simulink library "inp"  (Simulink 8.2) (continued)
== system inp/INP/InputSignals/DirectPassThrough == (continued)
  Cast230/1 -> Bus/69  "VINP_PassengerDoorOpenValid_flg"
  Bus Selector226/11 -> Cast229/1  "<VINP_PassengerDoorOpen_flg_InHAL>"
  Bus Selector226/12 -> Cast230/1  "<VINP_PassengerDoorOpenValid_flg_InHAL>"
  Cast231/1 -> Bus/70  "VINP_LRDoorOpen_flg"
  Cast232/1 -> Bus/71  "VINP_LRDoorOpenValid_flg"
  Bus Selector226/13 -> Cast231/1  "<VINP_LRDoorOpen_flg_InHAL>"
  Bus Selector226/14 -> Cast232/1  "<VINP_LRDoorOpenValid_flg_InHAL>"
  Cast4/1 -> Bus/72  "VINP_RRDoorOpen_flg"
  Cast5/1 -> Bus/73  "VINP_RRDoorOpenValid_flg"
  Bus Selector226/15 -> Cast4/1  "<VINP_RRDoorOpen_flg_InHAL>"
  Bus Selector226/16 -> Cast5/1  "<VINP_RRDoorOpenValid_flg_InHAL>"
  Cast13/1 -> Bus/74  "VINP_TailGateOpen_flg"
  Cast14/1 -> Bus/75  "VINP_TailGateOpenValid_flg"
  Bus Selector226/17 -> Cast13/1  "<VINP_TailGateOpen_flg_InHAL>"
  Bus Selector226/18 -> Cast14/1  "<VINP_TailGateOpenValid_flg_InHAL>"
  Cast15/1 -> Bus/76  "VINP_AnyDoorOpen_flg"
  Cast16/1 -> Bus/77  "VINP_AnyDoorOpenValid_flg"
  Bus Selector226/19 -> Cast15/1  "<VINP_AnyDoorOpen_flg_InHAL>"
  Bus Selector226/20 -> Cast16/1  "<VINP_AnyDoorOpenValid_flg_InHAL>"
  Cast17/1 -> Bus/78  "VINP_VehCrashed_flg"
  Cast18/1 -> Bus/79  "VINP_VehCrashedValid_flg"
  Bus Selector226/21 -> Cast17/1  "<VINP_VehCrashed_flg_InHAL>"
  Bus Selector226/22 -> Cast18/1  "<VINP_VehCrashedValid_flg_InHAL>"
  Cast19/1 -> Bus/96  "VINP_HSAActive_flg"
  Cast20/1 -> Bus/97  "VINP_HSAActiveValid_flg"
  Bus Selector209/17 -> Cast19/1  "<VINP_HSAActive_flg_InHAL>"
  Bus Selector209/18 -> Cast20/1  "<VINP_HSAActiveValid_flg_InHAL>"
  Cast21/1 -> Bus/98  "VINP_HSAFault_flg"
  Cast22/1 -> Bus/99  "VINP_HSAFaultValid_flg"
  Bus Selector209/19 -> Cast21/1  "<VINP_HSAFault_flg_InHAL>"
  Bus Selector209/20 -> Cast22/1  "<VINP_HSAFaultValid_flg_InHAL>"
  Cast23/1 -> Bus/113  "VINP_SurfaceGradeValid_flg"
  Cast24/1 -> Bus/112  "VINP_SurfaceGrade_deg"
  Bus Selector7/13 -> Cast24/1  "<VINP_SurfaceGrade_deg_InHAL>"
  Bus Selector7/14 -> Cast23/1  "<VINP_SurfaceGradeValid_flg_InHAL>"
  Cast25/1 -> Bus/115  "VINP_BrakBoosterPresValid_flg"
  Cast26/1 -> Bus/114  "VINP_BrakBoosterPres_kPa"
  Bus Selector7/15 -> Cast26/1  "<VINP_BrakBoosterPres_kPa_InHAL>"
  Bus Selector7/16 -> Cast25/1  "<VINP_BrakBoosterPresValid_flg_InHAL>"
  Cast27/1 -> Bus/116  "VINP_LowBrakBoosterPres_flg"
  Cast28/1 -> Bus/117  "VINP_LowBrakBoosterPresValid_flg"
  Bus Selector7/17 -> Cast27/1  "<VINP_LowBrakBoosterPres_flg_InHAL>"
  Bus Selector7/18 -> Cast28/1  "<VINP_LowBrakBoosterPresValid_flg_InHAL>"
  [ABS_signal_bus] -> Bus Selector3/1
  Cast34/1 -> Bus/119  "VINP_LFWheelSpeedValid_flg"
  Cast35/1 -> Bus/118  "VINP_LFWheelSpeed_kph"
  Bus Selector3/1 -> Cast35/1  "<VINP_LFWheelSpeed_kph_InHAL>"
  Bus Selector3/2 -> Cast34/1  "<VINP_LFWheelSpeedValid_flg_InHAL>"
  Cast36/1 -> Bus/121  "VINP_LRWheelSpeedValid_flg"
  Cast37/1 -> Bus/120  "VINP_LRWheelSpeed_kph"
  Bus Selector3/3 -> Cast37/1  "<VINP_LRWheelSpeed_kph_InHAL>"
  Bus Selector3/4 -> Cast36/1  "<VINP_LRWheelSpeedValid_flg_InHAL>"
  Cast38/1 -> Bus/123  "VINP_RFWheelSpeedValid_flg"
  Cast39/1 -> Bus/122  "VINP_RFWheelSpeed_kph"
  Bus Selector3/5 -> Cast39/1  "<VINP_RFWheelSpeed_kph_InHAL>"
  Bus Selector3/6 -> Cast38/1  "<VINP_RFWheelSpeedValid_flg_InHAL>"
  Cast40/1 -> Bus/125  "VINP_RRWheelSpeedValid_flg"
  Cast41/1 -> Bus/124  "VINP_RRWheelSpeed_kph"
  Bus Selector3/7 -> Cast41/1  "<VINP_RRWheelSpeed_kph_InHAL>"
  Bus Selector3/8 -> Cast40/1  "<VINP_RRWheelSpeedValid_flg_InHAL>"
  Cast42/1 -> Bus/131  "VINP_RelSteerAngleValid_flg"
  Cast44/1 -> Bus/130  "VINP_RelSteerAngle_deg"
  Bus Selector232/1 -> Cast44/1  "<VINP_RelSteerAngle_deg_InHAL>"
  Bus Selector232/2 -> Cast42/1  "<VINP_RelSteerAngleValid_flg_InHAL>"
  Cast151/1 -> Bus/136  "VINP_EPSFailed_flg"
  Cast152/1 -> Bus/137  "VINP_EPSFailedValid_flg"
  Bus Selector232/7 -> Cast151/1  "<VINP_EPSFailed_flg_InHAL>"
  Bus Selector232/8 -> Cast152/1  "<VINP_EPSFailedValid_flg_InHAL>"
  Cast153/1 -> Bus/139  "VINP_AckCurStrAngReqValid_flg"
  Cast154/1 -> Bus/138  "VINP_AckCurStrAngReq_deg"
  Bus Selector232/9 -> Cast154/1  "<VINP_AckCurStrAngReq_deg_InHAL>"
  Bus Selector232/10 -> Cast153/1  "<VINP_AckCurStrAngReqValid_flg_InHAL>"
  Cast155/1 -> Bus/140  "VINP_AckCurStrAngReqApplable_flg"
  Cast156/1 -> Bus/141  "VINP_AckCurStrAngReqApplableValid_flg"
  Bus Selector232/11 -> Cast155/1  "<VINP_AckCurStrAngReqApplable_flg_InHAL>"
  Bus Selector232/12 -> Cast156/1  "<VINP_AckCurStrAngReqApplableValid_flg_InHAL>"
  Cast157/1 -> Bus/143  "VINP_AckCurStrTqReqValid_flg"
  Cast158/1 -> Bus/142  "VINP_AckCurStrTqReq_Nm"
  Bus Selector232/13 -> Cast158/1  "<VINP_AckCurStrTqReq_Nm_InHAL>"
  Bus Selector232/14 -> Cast157/1  "<VINP_AckCurStrTqReqValid_flg_InHAL>"
  Cast159/1 -> Bus/144  "VINP_AckCurStrTqReqApplable_flg"
  Cast160/1 -> Bus/145  "VINP_AckCurStrTqReqApplableValid_flg"
  Bus Selector232/15 -> Cast159/1  "<VINP_AckCurStrTqReqApplable_flg_InHAL>"
  Bus Selector232/16 -> Cast160/1  "<VINP_AckCurStrTqReqApplableValid_flg_InHAL>"
  Cast161/1 -> Bus/147  "VINP_EPSBattVoltValid_flg"
  Cast162/1 -> Bus/146  "VINP_EPSBattVolt_V"
  Bus Selector232/17 -> Cast162/1  "<VINP_EPSBattVolt_V_InHAL>"
  Bus Selector232/18 -> Cast161/1  "<VINP_EPSBattVoltValid_flg_InHAL>"
  [METER_signal_bus] -> Bus Selector6/1
  Cast167/1 -> Bus/157  "VINP_OdoMeterValid_flg"
  Cast168/1 -> Bus/156  "VINP_OdoMeter_km"
  Bus Selector6/1 -> Cast168/1  "<VINP_OdoMeter_km_InHAL>"
  Bus Selector6/2 -> Cast167/1  "<VINP_OdoMeterValid_flg_InHAL>"
  Cast169/1 -> Bus/158  "VINP_LowBrakeFluid_flg"
  Cast170/1 -> Bus/159  "VINP_LowBrakeFluidValid_flg"
  Bus Selector6/3 -> Cast169/1  "<VINP_LowBrakeFluid_flg_InHAL>"
  Bus Selector6/4 -> Cast170/1  "<VINP_LowBrakeFluidValid_flg_InHAL>"
  Cast171/1 -> Bus/160  "VINP_ParkBrakeOn_flg"
  Cast172/1 -> Bus/161  "VINP_ParkBrakeOnValid_flg"
  Bus Selector6/5 -> Cast171/1  "<VINP_ParkBrakeOn_flg_InHAL>"
  Bus Selector6/6 -> Cast172/1  "<VINP_ParkBrakeOnValid_flg_InHAL>"
  Cast173/1 -> Bus/162  "VINP_SeatBeltNOTFasten_flg"
  Cast174/1 -> Bus/163  "VINP_SeatBeltNOTFastenValid_flg"
  Bus Selector6/7 -> Cast173/1  "<VINP_SeatBeltNOTFasten_flg_InHAL>"
  Bus Selector6/8 -> Cast174/1  "<VINP_SeatBeltNOTFastenValid_flg_InHAL>"
  Cast175/1 -> Bus/165  "VINP_MeterVehSpdValid_flg"
  Cast176/1 -> Bus/164  "VINP_MeterVehSpd_kph"
  Bus Selector6/9 -> Cast176/1  "<VINP_MeterVehSpd_kph_InHAL>"
  Bus Selector6/10 -> Cast175/1  "<VINP_MeterVehSpdValid_flg_InHAL>"
  [AC_signal_bus] -> Bus Selector8/1
  Cast177/1 -> Bus/167  "VINP_OutsideTempValid_flg"
  Cast178/1 -> Bus/166  "VINP_OutsideTemp_degC"
  Bus Selector8/1 -> Cast178/1  "<VINP_OutsideTemp_degC_InHAL>"
  Bus Selector8/2 -> Cast177/1  "<VINP_OutsideTempValid_flg_InHAL>"
  [EPB_signal_bus] -> Bus Selector9/1
  Bus Selector9/1 -> Cast179/1  "<VINP_ParkBrakeBindSta_enum_InHAL>"
  Bus Selector9/2 -> Cast180/1  "<VINP_ParkBrakeBindStaValid_flg_InHAL>"
  Cast179/1 -> Bus/168  "VINP_ParkBrakeBindSta_enum"
  Cast180/1 -> Bus/169  "VINP_ParkBrakeBindStaValid_flg"
  [VCU_signal_bus] -> Bus Selector10/1
  Cast233/1 -> Bus/174  "VINP_AckCurBrakDecReq_mps2"
  Cast234/1 -> Bus/175  "VINP_AckCurBrakDecReqValid_flg"
  Bus Selector10/1 -> Cast233/1  "<VINP_AckCurBrakDecReq_mps2_InHAL>"
  Bus Selector10/2 -> Cast234/1  "<VINP_AckCurBrakDecReqValid_flg_InHAL>"
  Cast235/1 -> Bus/176  "VINP_AckCurBrakDecReqApplable_flg"
  Cast236/1 -> Bus/177  "VINP_AckCurBrakDecReqApplableValid_flg"
  Bus Selector10/3 -> Cast235/1  "<VINP_AckCurBrakDecReqApplable_flg_InHAL>"
  Bus Selector10/4 -> Cast236/1  "<VINP_AckCurBrakDecReqApplableValid_flg_InHAL>"
  Cast237/1 -> Bus/178  "VINP_AckCurPTTqReq_Nm"
  Cast238/1 -> Bus/179  "VINP_AckCurPTTqReqValid_flg"
  Cast239/1 -> Bus/180  "VINP_AckCurPTTqReqApplable_flg"
  Cast240/1 -> Bus/181  "VINP_AckCurPTTqReqApplableValid_flg"
  Bus Selector10/5 -> Cast237/1  "<VINP_AckCurPTTqReq_Nm_InHAL>"
  Bus Selector10/6 -> Cast238/1  "<VINP_AckCurPTTqReqValid_flg_InHAL>"
  Bus Selector10/7 -> Cast239/1  "<VINP_AckCurPTTqReqApplable_flg_InHAL>"
  Bus Selector10/8 -> Cast240/1  "<VINP_AckCurPTTqReqApplableValid_flg_InHAL>"
  Bus Selector10/9 -> Cast241/1  "<VINP_AckCurVehDec_mps2_InHAL>"
  Cast241/1 -> Bus/182  "VINP_AckCurVehDec_mps2"
  Cast242/1 -> Bus/183  "VINP_AckCurVehDecValid_flg"
  Bus Selector10/10 -> Cast242/1  "<VINP_AckCurVehDecValid_flg_InHAL>"
  Bus Selector10/11 -> Cast245/1  "<VINP_DeceContManag_flg_InHAL>"
  Cast245/1 -> Bus/184  "VINP_DeceContManag_flg"
  Cast246/1 -> Bus/185  "VINP_DeceContManagValid_flg"
  Bus Selector10/12 -> Cast246/1  "<VINP_DeceContManagValid_flg_InHAL>"
  Bus Selector10/13 -> Cast247/1  "<VINP_DriverBrakPedalOn_flg_InHAL>"
  Cast247/1 -> Bus/186  "VINP_DriverBrakPedalOn_flg"
  Cast248/1 -> Bus/187  "VINP_DriverBrakPedalOnValid_flg"
  Bus Selector10/14 -> Cast248/1  "<VINP_DriverBrakPedalOnValid_flg_InHAL>"
  Bus Selector10/15 -> Cast249/1  "<VINP_ECMExtnTqAvailable_flg_InHAL>"
  Cast249/1 -> Bus/188  "VINP_ECMExtnTqAvailable_flg"
  Cast250/1 -> Bus/189  "VINP_ECMExtnTqAvailableValid_flg"
  Bus Selector10/16 -> Cast250/1  "<VINP_ECMExtnTqAvailableValid_flg_InHAL>"
  Bus Selector10/17 -> Cast251/1  "<VINP_ECMTqSource_enum_InHAL>"
  Cast251/1 -> Bus/190  "VINP_ECMTqSource_enum"
  Cast252/1 -> Bus/191  "VINP_ECMTqSourceValid_flg"
  Bus Selector10/18 -> Cast252/1  "<VINP_ECMTqSourceValid_flg_InHAL>"
  Bus Selector10/19 -> Cast253/1  "<VINP_ESCBrakeStatus_enum_InHAL>"
  Cast253/1 -> Bus/192  "VINP_ESCBrakeStatus_enum"
  Cast254/1 -> Bus/193  "VINP_ESCBrakeStatusValid_flg"
  Bus Selector10/20 -> Cast254/1  "<VINP_ESCBrakeStatusValid_flg_InHAL>"
  Bus Selector10/21 -> Cast255/1  "<VINP_ESCExtnBrkAvailable_flg_InHAL>"
  Cast255/1 -> Bus/194  "VINP_ESCExtnBrkAvailable_flg"
  Cast256/1 -> Bus/195  "VINP_ESCExtnBrkAvailableValid_flg"
  Bus Selector10/22 -> Cast256/1  "<VINP_ESCExtnBrkAvailableValid_flg_InHAL>"
  Bus Selector10/23 -> Cast257/1  "<VINP_ESCBattVolt_V_InHAL>"
  Cast257/1 -> Bus/196  "VINP_ESCBattVolt_V"
  Cast258/1 -> Bus/197  "VINP_ESCBattVoltValid_flg"
  Bus Selector10/24 -> Cast258/1  "<VINP_ESCBattVoltValid_flg_InHAL>"
  Bus Selector10/25 -> Cast259/1  "<VINP_HWAccelPedalPosn_pct_InHAL>"
  Cast259/1 -> Bus/198  "VINP_HWAccelPedalPosn_pct"
  Cast260/1 -> Bus/199  "VINP_HWAccelPedalPosnValid_flg"
  Bus Selector10/26 -> Cast260/1  "<VINP_HWAccelPedalPosnValid_flg_InHAL>"
  Cast263/1 -> Bus/208  "VINP_HCUMILReq_flg"
  Cast264/1 -> Bus/209  "VINP_HCUMILReqValid_flg"
  [VCU_signal_bus] -> Bus Selector11/1
  Cast271/1 -> Bus/218  "VINP_ACCTimeGapIncVCU_flg"
  Cast272/1 -> Bus/219  "VINP_ACCTimeGapIncVCUValid_flg"
  Bus Selector11/1 -> Cast271/1  "<VINP_ACCTimeGapIncVCU_flg_InHAL>"
  Bus Selector11/2 -> Cast272/1  "<VINP_ACCTimeGapIncVCUValid_flg_InHAL>"
  Cast273/1 -> Bus/220  "VINP_ACCTimeGapDecVCU_flg"
  Cast274/1 -> Bus/221  "VINP_ACCTimeGapDecVCUValid_flg"
  Bus Selector11/3 -> Cast273/1  "<VINP_ACCTimeGapDecVCU_flg_InHAL>"
  Bus Selector11/4 -> Cast274/1  "<VINP_ACCTimeGapDecVCUValid_flg_InHAL>"
  Cast275/1 -> Bus/222  "VINP_LFCOnVCU_flg"
  Cast276/1 -> Bus/223  "VINP_LFCOnVCUValid_flg"
  [HMI_signal_bus] -> Bus Selector12/1
  Cast277/1 -> Bus/226  "VINP_AEBEnableH_flg"
  Cast278/1 -> Bus/227  "VINP_AEBEnableHValid_flg"
  Bus Selector12/1 -> Cast277/1  "<VINP_AEBEnableH_flg_InHAL>"
  Bus Selector12/2 -> Cast278/1  "<VINP_AEBEnableHValid_flg_InHAL>"
  Cast279/1 -> Bus/228  "VINP_FCWEnableH_flg"
  Cast280/1 -> Bus/229  "VINP_FCWEnableHValid_flg"
  Bus Selector12/3 -> Cast279/1  "<VINP_FCWEnableH_flg_InHAL>"
  Bus Selector12/4 -> Cast280/1  "<VINP_FCWEnableHValid_flg_InHAL>"
  Cast281/1 -> Bus/230  "VINP_CSCEnableH_flg"
  Bus Selector12/5 -> Cast281/1  "<VINP_CSCEnableH_flg_InHAL>"
  Cast282/1 -> Bus/231  "VINP_CSCEnableHValid_flg"
  Bus Selector12/6 -> Cast282/1  "<VINP_CSCEnableHValid_flg_InHAL>"
  Cast283/1 -> Bus/232  "VINP_LKAEnableH_flg"
  Cast284/1 -> Bus/233  "VINP_LKAEnableHValid_flg"
  Bus Selector12/7 -> Cast283/1  "<VINP_LKAEnableH_flg_InHAL>"
  Bus Selector12/8 -> Cast284/1  "<VINP_LKAEnableHValid_flg_InHAL>"
  Cast285/1 -> Bus/234  "VINP_MAPAEnableH_flg"
  Cast286/1 -> Bus/235  "VINP_MAPAEnableHValid_flg"
  Bus Selector12/9 -> Cast285/1  "<VINP_MAPAEnableH_flg_InHAL>"
  Bus Selector12/10 -> Cast286/1  "<VINP_MAPAEnableHValid_flg_InHAL>"
  Cast287/1 -> Bus/236  "VINP_LCSEnableH_flg"
  Cast288/1 -> Bus/237  "VINP_LCSEnableHValid_flg"
  Bus Selector12/11 -> Cast287/1  "<VINP_LCSEnableH_flg_InHAL>"
  Bus Selector12/12 -> Cast288/1  "<VINP_LCSEnableHValid_flg_InHAL>"
  [HMI_signal_bus] -> Bus Selector13/1
  [INP_calc_bus] -> Bus Selector14/1
  Cast289/1 -> Bus/242  "VINP_LFCPilotOnH_flg"
  Cast290/1 -> Bus/243  "VINP_LFCPilotOnHValid_flg"
  Bus Selector14/1 -> Cast289/1  "<VINP_LFCPilotOnH_flg_InProc>"
  Bus Selector13/1 -> Cast290/1  "<VINP_LFCPilotOnHValid_flg_InHAL>"
  [HMI_signal_bus] -> Bus Selector15/1
  [INP_calc_bus] -> Bus Selector16/1
  Cast291/1 -> Bus/244  "VINP_LFCPilotCancelH_flg"
  Cast292/1 -> Bus/245  "VINP_LFCPilotCancelHValid_flg"
  Bus Selector16/1 -> Cast291/1  "<VINP_LFCPilotCancelH_flg_InProc>"
  Bus Selector15/1 -> Cast292/1  "<VINP_LFCPilotCancelHValid_flg_InHAL>"
  [HMI_signal_bus] -> Bus Selector17/1
  [INP_calc_bus] -> Bus Selector18/1
  Cast293/1 -> Bus/246  "VINP_ACCOnH_flg"
  Cast294/1 -> Bus/247  "VINP_ACCOnHValid_flg"
  Bus Selector18/1 -> Cast293/1  "<VINP_ACCOnH_flg_InProc>"
  Bus Selector17/1 -> Cast294/1  "<VINP_ACCOnHValid_flg_InHAL>"
  [HMI_signal_bus] -> Bus Selector19/1
  [INP_calc_bus] -> Bus Selector20/1
  Cast295/1 -> Bus/248  "VINP_ACCCancelH_flg"
  Cast296/1 -> Bus/249  "VINP_ACCCancelHValid_flg"
  Bus Selector20/1 -> Cast295/1  "<VINP_ACCCancelH_flg_InProc>"
  Bus Selector19/1 -> Cast296/1  "<VINP_ACCCancelHValid_flg_InHAL>"
  [HMI_signal_bus] -> Bus Selector21/1
  [INP_calc_bus] -> Bus Selector22/1
  Cast297/1 -> Bus/250  "VINP_ACCResSpdIncH_flg"
  Cast298/1 -> Bus/251  "VINP_ACCResSpdIncHValid_flg"
  Bus Selector22/1 -> Cast297/1  "<VINP_ACCResSpdIncH_flg_InProc>"
  Bus Selector21/1 -> Cast298/1  "<VINP_ACCResSpdIncHValid_flg_InHAL>"
  [HMI_signal_bus] -> Bus Selector23/1
  [INP_calc_bus] -> Bus Selector24/1
  Cast299/1 -> Bus/252  "VINP_ACCSetSpdDecH_flg"
  Cast300/1 -> Bus/253  "VINP_ACCSetSpdDecHValid_flg"
  Bus Selector24/1 -> Cast299/1  "<VINP_ACCSetSpdDecH_flg_InProc>"
  Bus Selector23/1 -> Cast300/1  "<VINP_ACCSetSpdDecHValid_flg_InHAL>"
  [HMI_signal_bus] -> Bus Selector25/1
  [INP_calc_bus] -> Bus Selector26/1
  Cast301/1 -> Bus/254  "VINP_ACCSpdIncH_flg"
  Cast302/1 -> Bus/255  "VINP_ACCSpdIncHValid_flg"
  Bus Selector26/1 -> Cast301/1  "<VINP_ACCSpdIncH_flg_InProc>"
  Bus Selector25/1 -> Cast302/1  "<VINP_ACCSpdIncHValid_flg_InHAL>"
  [HMI_signal_bus] -> Bus Selector27/1
  [INP_calc_bus] -> Bus Selector28/1
  Cast303/1 -> Bus/256  "VINP_ACCSpdDecH_flg"
  Cast304/1 -> Bus/257  "VINP_ACCSpdDecHValid_flg"
  Bus Selector28/1 -> Cast303/1  "<VINP_ACCSpdDecH_flg_InProc>"
  Bus Selector27/1 -> Cast304/1  "<VINP_ACCSpdDecHValid_flg_InHAL>"
  [HMI_signal_bus] -> Bus Selector29/1
  [INP_calc_bus] -> Bus Selector30/1
  Cast305/1 -> Bus/258  "VINP_ACCTimeGapIncH_flg"
  Cast306/1 -> Bus/259  "VINP_ACCTimeGapIncHValid_flg"
  Bus Selector30/1 -> Cast305/1  "<VINP_ACCTimeGapIncH_flg_InProc>"
  Bus Selector29/1 -> Cast306/1  "<VINP_ACCTimeGapIncHValid_flg_InHAL>"
  [HMI_signal_bus] -> Bus Selector31/1
  [INP_calc_bus] -> Bus Selector32/1
  Cast307/1 -> Bus/260  "VINP_ACCTimeGapDecH_flg"
  Cast308/1 -> Bus/261  "VINP_ACCTimeGapDecHValid_flg"
  Bus Selector32/1 -> Cast307/1  "<VINP_ACCTimeGapDecH_flg_InProc>"
  Bus Selector31/1 -> Cast308/1  "<VINP_ACCTimeGapDecHValid_flg_InHAL>"
  [HMI_signal_bus] -> Bus Selector33/1
  [INP_calc_bus] -> Bus Selector34/1
  Cast309/1 -> Bus/262  "VINP_IGSAOnH_flg"
  Cast310/1 -> Bus/263  "VINP_IGSAOnHValid_flg"
  Bus Selector34/1 -> Cast309/1  "<VINP_IGSAOnH_flg_InProc>"
  Bus Selector33/1 -> Cast310/1  "<VINP_IGSAOnHValid_flg_InHAL>"
  [HMI_signal_bus] -> Bus Selector35/1
  [INP_calc_bus] -> Bus Selector36/1
  Cast311/1 -> Bus/264  "VINP_IGSAResSpdIncH_flg"
  Cast312/1 -> Bus/265  "VINP_IGSAResSpdIncHValid_flg"
  Bus Selector36/1 -> Cast311/1  "<VINP_IGSAResSpdIncH_flg_InProc>"
  Bus Selector35/1 -> Cast312/1  "<VINP_IGSAResSpdIncHValid_flg_InHAL>"
  [HMI_signal_bus] -> Bus Selector37/1
  [INP_calc_bus] -> Bus Selector38/1
  Cast313/1 -> Bus/266  "VINP_IGSASetSpdDecH_flg"
  Cast314/1 -> Bus/267  "VINP_IGSASetSpdDecHValid_flg"
  Bus Selector38/1 -> Cast313/1  "<VINP_IGSASetSpdDecH_flg_InProc>"
  Bus Selector37/1 -> Cast314/1  "<VINP_IGSASetSpdDecHValid_flg_InHAL>"
  [HMI_signal_bus] -> Bus Selector39/1
  [INP_calc_bus] -> Bus Selector40/1
  Cast315/1 -> Bus/268  "VINP_IGSASwitchModeH_flg"
  Cast316/1 -> Bus/269  "VINP_IGSASwitchModeHValid_flg"
  Bus Selector40/1 -> Cast315/1  "<VINP_IGSASwitchModeH_flg_InProc>"
  Bus Selector39/1 -> Cast316/1  "<VINP_IGSASwitchModeHValid_flg_InHAL>"
  Cast64/1 -> Bus/442  "VINP_ABSCANValid_flg"
  Cast65/1 -> Bus/454  "VINP_ABSCANAlive_flg"
  Cast68/1 -> Bus/443  "VINP_BCMCANValid_flg"
  Cast69/1 -> Bus/455  "VINP_BCMCANAlive_flg"
  Cast321/1 -> Bus/444  "VINP_ECMCANValid_flg"
  Cast322/1 -> Bus/456  "VINP_ECMCANAlive_flg"
  Cast323/1 -> Bus/445  "VINP_TCMCANValid_flg"
  Cast324/1 -> Bus/457  "VINP_TCMCANAlive_flg"
  Cast325/1 -> Bus/446  "VINP_EPSCANValid_flg"
  Cast326/1 -> Bus/458  "VINP_EPSCANAlive_flg"
  [INP_diag_bus] -> Bus Selector241/1
  Bus Selector241/1 -> Cast64/1  "<VINP_ABSCANValid_flg_InProc>"
  [INP_diag_bus] -> Bus Selector242/1
  Bus Selector242/1 -> Cast65/1  "<VINP_ABSCANAlive_flg_InProc>"
  Bus Selector241/2 -> Cast68/1  "<VINP_BCMCANValid_flg_InProc>"
  Bus Selector242/2 -> Cast69/1  "<VINP_BCMCANAlive_flg_InProc>"
  Bus Selector241/3 -> Cast321/1  "<VINP_ECMCANValid_flg_InProc>"
  Bus Selector242/3 -> Cast322/1  "<VINP_ECMCANAlive_flg_InProc>"
  Bus Selector241/4 -> Cast323/1  "<VINP_TCMCANValid_flg_InProc>"
  Bus Selector242/4 -> Cast324/1  "<VINP_TCMCANAlive_flg_InProc>"
  Bus Selector241/5 -> Cast325/1  "<VINP_EPSCANValid_flg_InProc>"
  Bus Selector242/5 -> Cast326/1  "<VINP_EPSCANAlive_flg_InProc>"
  Cast56/1 -> Bus/447  "VINP_METERCANValid_flg"
  Bus Selector241/6 -> Cast56/1  "<VINP_METERCANValid_flg_InProc>"
  Cast57/1 -> Bus/448  "VINP_ACCANValid_flg"
  Bus Selector241/7 -> Cast57/1  "<VINP_ACCANValid_flg_InProc>"
  Cast58/1 -> Bus/449  "VINP_EPBCANValid_flg"
  Bus Selector241/8 -> Cast58/1  "<VINP_EPBCANValid_flg_InProc>"
  Cast59/1 -> Bus/450  "VINP_VCUCANValid_flg"
  Bus Selector241/9 -> Cast59/1  "<VINP_VCUCANValid_flg_InProc>"
  Cast60/1 -> Bus/459  "VINP_METERCANAlive_flg"
  Bus Selector242/6 -> Cast60/1  "<VINP_METERCANAlive_flg_InProc>"
  Cast61/1 -> Bus/460  "VINP_ACCANAlive_flg"
  Bus Selector242/7 -> Cast61/1  "<VINP_ACCANAlive_flg_InProc>"
  Cast62/1 -> Bus/461  "VINP_EPBCANAlive_flg"
  Bus Selector242/8 -> Cast62/1  "<VINP_EPBCANAlive_flg_InProc>"
  Cast63/1 -> Bus/462  "VINP_VCUCANAlive_flg"
  Bus Selector242/9 -> Cast63/1  "<VINP_VCUCANAlive_flg_InProc>"
  Cast327/1 -> Bus/451  "VINP_HMICANValid_flg"
  Bus Selector241/10 -> Cast327/1  "<VINP_HMICANValid_flg_InProc>"
  Cast328/1 -> Bus/453  "VINP_EPMCANValid_flg"
  Bus Selector241/12 -> Cast328/1  "<VINP_EPMCANValid_flg_InProc>"
  Cast329/1 -> Bus/463  "VINP_HMICANAlive_flg"
  Bus Selector242/10 -> Cast329/1  "<VINP_HMICANAlive_flg_InProc>"
  Cast330/1 -> Bus/465  "VINP_EPMCANAlive_flg"
  Bus Selector242/12 -> Cast330/1  "<VINP_EPMCANAlive_flg_InProc>"
  Cast331/1 -> Bus/3  "VINP_BatteryVolt_V"
  Cast332/1 -> Bus/4  "VINP_BatteryVoltValid_flg"
  Bus Selector197/3 -> Cast331/1  "<VINP_BatteryVolt_V_InHAL>"
  Bus Selector197/4 -> Cast332/1  "<VINP_BatteryVoltValid_flg_InHAL>"
  Cast334/1 -> Bus/129  "VINP_SteerSpeedValid_flg"
  Cast333/1 -> Bus/128  "VINP_SteerSpeed_degps"
  Bus Selector42/1 -> Cast333/1  "<VINP_SteerSpeed_degps_InProc>"
  Bus Selector42/2 -> Cast334/1  "<VINP_SteerSpeedValid_flg_InProc>"
  Cast335/1 -> Bus/148  "VINP_EPASMILReq_flg"
  Bus Selector232/19 -> Cast335/1  "<VINP_EPASMILReq_flg_InHAL>"
  Cast336/1 -> Bus/149  "VINP_EPASMILReqValid_flg"
  Bus Selector232/20 -> Cast336/1  "<VINP_EPASMILReqValid_flg_InHAL>"
  Cast337/1 -> Bus/200  "VINP_CurDriverTqReq_Nm"
  Cast338/1 -> Bus/201  "VINP_CurDriverTqReqValid_flg"
  Bus Selector10/27 -> Cast337/1  "<VINP_CurDriverTqReq_Nm_InHAL>"
  Bus Selector10/28 -> Cast338/1  "<VINP_CurDriverTqReqValid_flg_InHAL>"
  Cast339/1 -> Bus/238  "VINP_FCWWarningModeH_enum"
  Cast340/1 -> Bus/239  "VINP_FCWWarningModeHValid_flg"
  Bus Selector12/13 -> Cast339/1  "<VINP_FCWWarningModeH_enum_InHAL>"
  Bus Selector12/14 -> Cast340/1  "<VINP_FCWWarningModeHValid_flg_InHAL>"
  [EPS_signal_bus] -> Bus Selector41/1
  [INP_calc_bus] -> Bus Selector42/1
  Cast341/1 -> Bus/224  "VINP_MSAOnVCU_flg"
  Cast342/1 -> Bus/225  "VINP_MSAOnVCUValid_flg"
  Cast343/1 -> Bus/240  "VINP_DefMaxACCSetSpeed_kph"
  Cast344/1 -> Bus/241  "VINP_DefMaxACCSetSpeedValid_flg"
  Bus Selector12/15 -> Cast343/1  "<VINP_DefMaxACCSetSpeed_kph_InHAL>"
  Bus Selector12/16 -> Cast344/1  "<VINP_DefMaxACCSetSpeedValid_flg_InHAL>"
  [CSW_signal_bus] -> Terminator13/1
  CSW_signal_bus/1 -> [CSW_signal_bus]
  Bus Selector204/25 -> Cast1/1  "<VINP_OBDWarnCycAchiv_flg_InHAL>"
  Cast1/1 -> Bus/36  "VINP_OBDWarnCycAchiv_flg"
  Cast66/1 -> Bus/37  "VINP_OBDWarnCycAchivValid_flg"
  Bus Selector204/26 -> Cast66/1  "<VINP_OBDWarnCycAchivValid_flg_InHAL>"
  Cast67/1 -> Bus/38  "VINP_OBDDriveCycAchiv_flg"
  Cast78/1 -> Bus/39  "VINP_OBDDriveCycAchivValid_flg"
  Bus Selector204/27 -> Cast67/1  "<VINP_OBDDriveCycAchiv_flg_InHAL>"
  Bus Selector204/28 -> Cast78/1  "<VINP_OBDDriveCycAchivValid_flg_InHAL>"
  Bus Selector230/9 -> Cast141/1  "<VINP_TqConvClutchSt_enum_InHAL>"
  Cast141/1 -> Bus/48  "VINP_TqConvClutchSt_enum"
  Cast142/1 -> Bus/49  "VINP_TqConvClutchStValid_flg"
  Bus Selector230/10 -> Cast142/1  "<VINP_TqConvClutchStValid_flg_InHAL>"
  Bus Selector230/11 -> Cast182/1  "<VINP_TCMMILReq_flg_InHAL>"
  Cast182/1 -> Bus/50  "VINP_TCMMILReq_flg"
  Cast184/1 -> Bus/51  "VINP_TCMMILReqValid_flg"
  Bus Selector230/12 -> Cast184/1  "<VINP_TCMMILReqValid_flg_InHAL>"
  Bus Selector230/13 -> Cast185/1  "<VINP_TCMWinterMode_flg_InHAL>"
  Cast185/1 -> Bus/52  "VINP_TCMWinterMode_flg"
  Cast200/1 -> Bus/53  "VINP_TCMWinterModeValid_flg"
  Bus Selector230/14 -> Cast200/1  "<VINP_TCMWinterModeValid_flg_InHAL>"
  Cast201/1 -> Bus/54  "VINP_TCMPowerMode_flg"
  Cast224/1 -> Bus/55  "VINP_TCMPowerModeValid_flg"
  Bus Selector230/15 -> Cast201/1  "<VINP_TCMPowerMode_flg_InHAL>"
  Bus Selector230/16 -> Cast224/1  "<VINP_TCMPowerModeValid_flg_InHAL>"
  Cast30/1 -> Bus/150  "VINP_EPSLKAStatus_enum"
  Bus Selector232/21 -> Cast30/1  "<VINP_EPSLKAStatus_enum_InHAL>"
  Cast32/1 -> Bus/151  "VINP_EPSLKAStatusValid_flg"
  Bus Selector232/22 -> Cast32/1  "<VINP_EPSLKAStatusValid_flg_InHAL>"
  Cast163/1 -> Bus/152  "VINP_EPSLFCStatus_enum"
  Cast164/1 -> Bus/153  "VINP_EPSLFCStatusValid_flg"
  Bus Selector232/23 -> Cast163/1  "<VINP_EPSLFCStatus_enum_InHAL>"
  Bus Selector232/24 -> Cast164/1  "<VINP_EPSLFCStatusValid_flg_InHAL>"
  Cast165/1 -> Bus/154  "VINP_EPSADASAbReason_enum"
  Cast166/1 -> Bus/155  "VINP_EPSADASAbReasonValid_flg"
  Bus Selector232/25 -> Cast165/1  "<VINP_EPSADASAbReason_enum_InHAL>"
  Bus Selector232/26 -> Cast166/1  "<VINP_EPSADASAbReasonValid_flg_InHAL>"
  [CSW_signal_bus] -> Bus Selector43/1
  Cast225/1 -> Bus/170  "VINP_RearFogSwOn_flg"
  Cast345/1 -> Bus/171  "VINP_RearFogSwOnValid_flg"
  Cast346/1 -> Bus/172  "VINP_HighBeamSwOn_flg"
  Cast347/1 -> Bus/173  "VINP_HighBeamSwOnValid_flg"
  Bus Selector43/1 -> Cast346/1  "<VINP_HighBeamSwOn_flg_InHAL>"
  Bus Selector43/2 -> Cast347/1  "<VINP_HighBeamSwOnValid_flg_InHAL>"
  Bus Selector10/35 -> Cast263/1  "<VINP_HCUMILReq_flg_InHAL>"
  Bus Selector10/36 -> Cast264/1  "<VINP_HCUMILReqValid_flg_InHAL>"
  Bus Selector10/29 -> Cast243/1  "<VINP_TCMGearPosnReqAllow_enum_InHAL>"
  Cast243/1 -> Bus/202  "VINP_TCMGearPosnReqAllow_enum"
  Cast244/1 -> Bus/203  "VINP_TCMGearPosnReqAllowValid_flg"
  Bus Selector10/30 -> Cast244/1  "<VINP_TCMGearPosnReqAllowValid_flg_InHAL>"
  Cast261/1 -> Bus/204  "VINP_MSAStatus_enum"
  Cast262/1 -> Bus/205  "VINP_MSAStatusValid_flg"
  Bus Selector10/31 -> Cast261/1  "<VINP_MSAStatus_enum_InHAL>"
  Bus Selector10/32 -> Cast262/1  "<VINP_MSAStatusValid_flg_InHAL>"
  Cast348/1 -> Bus/206  "VINP_HybridLimpHome_flg"
  Cast349/1 -> Bus/207  "VINP_HybridLimpHomeValid_flg"
  Bus Selector10/33 -> Cast348/1  "<VINP_HybridLimpHome_flg_InHAL>"
  Bus Selector10/34 -> Cast349/1  "<VINP_HybridLimpHomeValid_flg_InHAL>"
  [VCU_signal_bus] -> Bus Selector44/1
  Cast350/1 -> Bus/210  "VINP_ACCOnVCU_flg"
  Cast351/1 -> Bus/211  "VINP_ACCOnVCUValid_flg"
  Bus Selector45/1 -> Cast350/1  "<VINP_ACCOnVCU_flg_InProc>"
  Bus Selector45/2 -> Cast351/1  "<VINP_ACCOnVCUValid_flg_InProc>"
  Cast352/1 -> Bus/212  "VINP_ACCResSpdIncVCU_flg"
  Cast353/1 -> Bus/213  "VINP_ACCResSpdIncVCUValid_flg"
  Bus Selector44/1 -> Cast352/1  "<VINP_ACCResSpdIncVCU_flg_InHAL>"
  Bus Selector44/2 -> Cast353/1  "<VINP_ACCResSpdIncVCUValid_flg_InHAL>"
  Cast354/1 -> Bus/214  "VINP_ACCSetSpdDecVCU_flg"
  Cast355/1 -> Bus/215  "VINP_ACCSetSpdDecVCUValid_flg"
  Bus Selector44/3 -> Cast354/1  "<VINP_ACCSetSpdDecVCU_flg_InHAL>"
  Bus Selector44/4 -> Cast355/1  "<VINP_ACCSetSpdDecVCUValid_flg_InHAL>"
  Cast356/1 -> Bus/216  "VINP_ACCDistVCU_flg"
  Cast357/1 -> Bus/217  "VINP_ACCDistVCUValid_flg"
  Bus Selector46/1 -> Cast356/1  "<VINP_ACCDistVCU_flg_InProc>"
  Bus Selector46/2 -> Cast357/1  "<VINP_ACCDistVCUValid_flg_InProc>"
  [INP_calc_bus] -> Bus Selector45/1
  [INP_calc_bus] -> Bus Selector46/1
  Cast265/1 -> Bus/308  "VINP_ViewRangeEndR_m"
  Cast266/1 -> Bus/309  "VINP_ViewRangeEndRValid_flg"
  Bus Selector5/19 -> Cast265/1  "<VINP_ViewRangeEndR_m_InHAL>"
  Bus Selector5/20 -> Cast266/1  "<VINP_ViewRangeEndRValid_flg_InHAL>"
  Cast267/1 -> Bus/306  "VINP_ViewRangeEndL_m"
  Cast268/1 -> Bus/307  "VINP_ViewRangeEndLValid_flg"
  Bus Selector5/17 -> Cast267/1  "<VINP_ViewRangeEndL_m_InHAL>"
  Bus Selector5/18 -> Cast268/1  "<VINP_ViewRangeEndLValid_flg_InHAL>"
  [EPM_signal_bus] -> Bus Selector47/1
  Bus Selector47/1 -> Cast269/1  "<VINP_CamNofObjects_num_InHAL>"
  Cast269/1 -> Bus/354  "VINP_CamNofObjects_num"
  Cast270/1 -> Bus/355  "VINP_CamNofObjectsValid_flg"
  Bus Selector47/2 -> Cast270/1  "<VINP_CamNofObjectsValid_flg_InHAL>"
  Bus Selector47/3 -> Cast317/1  "<VINP_CamGoDecision_enum_InHAL>"
  Cast317/1 -> Bus/356  "VINP_CamGoDecision_enum"
  Cast318/1 -> Bus/357  "VINP_CamGoDecisionValid_flg"
  Bus Selector47/4 -> Cast318/1  "<VINP_CamGoDecisionValid_flg_InHAL>"
  Bus Selector47/5 -> Cast319/1  "<VINP_CloseCarDetected_flg_InHAL>"
  Cast319/1 -> Bus/358  "VINP_CloseCarDetected_flg"
  Cast320/1 -> Bus/359  "VINP_CloseCarDetectedValid_flg"
  Bus Selector47/6 -> Cast320/1  "<VINP_CloseCarDetectedValid_flg_InHAL>"
  Cast358/1 -> Bus/360  "VINP_RightCarCutIn_flg"
  Cast359/1 -> Bus/361  "VINP_RightCarCutInValid_flg"
  Bus Selector47/7 -> Cast358/1  "<VINP_RightCarCutIn_flg_InHAL>"
  Bus Selector47/8 -> Cast359/1  "<VINP_RightCarCutInValid_flg_InHAL>"
  Cast360/1 -> Bus/362  "VINP_LeftCarCutIn_flg"
  Cast361/1 -> Bus/363  "VINP_LeftCarCutInValid_flg"
  Bus Selector47/9 -> Cast360/1  "<VINP_LeftCarCutIn_flg_InHAL>"
  Bus Selector47/10 -> Cast361/1  "<VINP_LeftCarCutInValid_flg_InHAL>"
  [EPM_signal_bus] -> Bus Selector48/1
  Cast362/1 -> Bus/364  "VINP_CamFSImpairedVD_flg"
  Cast363/1 -> Bus/365  "VINP_CamFSImpairedVDValid_flg"
  Bus Selector48/1 -> Cast362/1  "<VINP_CamFSImpairedVD_flg_InHAL>"
  Bus Selector48/2 -> Cast363/1  "<VINP_CamFSImpairedVDValid_flg_InHAL>"
  Cast364/1 -> Bus/366  "VINP_CamFSLowSun_flg"
  Cast365/1 -> Bus/367  "VINP_CamFSLowSunValid_flg"
  Bus Selector48/3 -> Cast364/1  "<VINP_CamFSLowSun_flg_InHAL>"
  Bus Selector48/4 -> Cast365/1  "<VINP_CamFSLowSunValid_flg_InHAL>"
  Cast366/1 -> Bus/368  "VINP_CamFSBlurImage_flg"
  Cast367/1 -> Bus/369  "VINP_CamFSBlurImageValid_flg"
  Bus Selector48/5 -> Cast366/1  "<VINP_CamFSBlurImage_flg_InHAL>"
  Bus Selector48/6 -> Cast367/1  "<VINP_CamFSBlurImageValid_flg_InHAL>"
  Cast368/1 -> Bus/370  "VINP_CamFSSmearImage_flg"
  Cast369/1 -> Bus/371  "VINP_CamFSSmearImageValid_flg"
  Bus Selector48/7 -> Cast368/1  "<VINP_CamFSSmearImage_flg_InHAL>"
  Bus Selector48/8 -> Cast369/1  "<VINP_CamFSSmearImageValid_flg_InHAL>"
  Cast370/1 -> Bus/372  "VINP_CamFSPartialBlockage_flg"
  Cast371/1 -> Bus/373  "VINP_CamFSPartialBlockageValid_flg"
  Bus Selector48/9 -> Cast370/1  "<VINP_CamFSPartialBlockage_flg_InHAL>"
  Bus Selector48/10 -> Cast371/1  "<VINP_CamFSPartialBlockageValid_flg_InHAL>"
  Cast372/1 -> Bus/374  "VINP_CamFSFullBlockage_flg"
  Cast373/1 -> Bus/375  "VINP_CamFSFullBlockageValid_flg"
  Bus Selector48/11 -> Cast372/1  "<VINP_CamFSFullBlockage_flg_InHAL>"
  Bus Selector48/12 -> Cast373/1  "<VINP_CamFSFullBlockageValid_flg_InHAL>"
  Cast374/1 -> Bus/376  "VINP_CamFSSplashes_flg"
  Cast375/1 -> Bus/377  "VINP_CamFSSplashesValid_flg"
  Bus Selector48/13 -> Cast374/1  "<VINP_CamFSSplashes_flg_InHAL>"
  Bus Selector48/14 -> Cast375/1  "<VINP_CamFSSplashesValid_flg_InHAL>"
  Cast376/1 -> Bus/378  "VINP_CamFSOutofCal_flg"
  Cast377/1 -> Bus/379  "VINP_CamFSOutofCalValid_flg"
  Bus Selector48/15 -> Cast376/1  "<VINP_CamFSOutofCal_flg_InHAL>"
  Bus Selector48/16 -> Cast377/1  "<VINP_CamFSOutofCalValid_flg_InHAL>"
  Cast378/1 -> Bus/380  "VINP_CamFSCalMisalign_flg"
  Cast379/1 -> Bus/381  "VINP_CamFSCalMisalignValid_flg"
  Bus Selector48/17 -> Cast378/1  "<VINP_CamFSCalMisalign_flg_InHAL>"
  Bus Selector48/18 -> Cast379/1  "<VINP_CamFSCalMisalignValid_flg_InHAL>"
  Cast380/1 -> Bus/382  "VINP_CamFSSmearedspots_flg"
  Cast381/1 -> Bus/383  "VINP_CamFSSmearedspotsValid_flg"
  Bus Selector48/19 -> Cast380/1  "<VINP_CamFSSmearedspots_flg_InHAL>"
  Bus Selector48/20 -> Cast381/1  "<VINP_CamFSSmearedspotsValid_flg_InHAL>"
  Cast382/1 -> Bus/384  "VINP_CamFSSpotHalosFrozen_flg"
  Cast383/1 -> Bus/385  "VINP_CamFSSpotHalosFrozenValid_flg"
  Bus Selector48/21 -> Cast382/1  "<VINP_CamFSSpotHalosFrozen_flg_InHAL>"
  Bus Selector48/22 -> Cast383/1  "<VINP_CamFSSpotHalosFrozenValid_flg_InHAL>"
  Cast384/1 -> Bus/386  "VINP_CamFSFoggySpots_flg"
  Cast385/1 -> Bus/387  "VINP_CamFSFoggySpotsValid_flg"
  Bus Selector48/23 -> Cast384/1  "<VINP_CamFSFoggySpots_flg_InHAL>"
  Bus Selector48/24 -> Cast385/1  "<VINP_CamFSFoggySpotsValid_flg_InHAL>"
  Cast386/1 -> Bus/388  "VINP_CamFSSunRay_flg"
  Cast387/1 -> Bus/389  "VINP_CamFSSunRayValid_flg"
  Bus Selector48/25 -> Cast386/1  "<VINP_CamFSSunRay_flg_InHAL>"
  Bus Selector48/26 -> Cast387/1  "<VINP_CamFSSunRayValid_flg_InHAL>"
  Cast388/1 -> Bus/452  "VINP_CSWCANValid_flg"
  Bus Selector241/11 -> Cast388/1  "<VINP_CSWCANValid_flg_InProc>"
  Cast389/1 -> Bus/464  "VINP_CSWCANAlive_flg"
  Bus Selector242/11 -> Cast389/1  "<VINP_CSWCANAlive_flg_InProc>"
  Bus Selector49/1 -> Cast225/1  "<VINP_RearFogSwOn_flg_InProc>"
  Bus Selector49/2 -> Cast345/1  "<VINP_RearFogSwOnValid_flg_InProc>"
  [INP_calc_bus] -> Bus Selector49/1
  Bus Selector50/1 -> Cast275/1  "<VINP_LFCOnVCU_flg_InProc>"
  Bus Selector50/2 -> Cast276/1  "<VINP_LFCOnVCUValid_flg_InProc>"
  [INP_calc_bus] -> Bus Selector50/1
  [VCU_signal_bus] -> Bus Selector51/1
  Bus Selector51/1 -> Cast341/1  "<VINP_MSAOnVCU_flg_InHAL>"
  Bus Selector51/2 -> Cast342/1  "<VINP_MSAOnVCUValid_flg_InHAL>"
  [INP_calc_bus] -> Bus Selector52/1
  Cast390/1 -> Bus/390  "VINP_CamObsID10_num"
  Cast391/1 -> Bus/391  "VINP_CamObsIDValid10_flg"
  Bus Selector52/1 -> Cast390/1  "<VINP_CamObsID10_num_InProc>"
  Bus Selector52/2 -> Cast391/1  "<VINP_CamObsIDValid10_flg_InProc>"
  Bus Selector52/3 -> Cast392/1  "<VINP_CamObsPosX10_m_InProc>"
  Cast392/1 -> Bus/392  "VINP_CamObsPosX10_m"
  Cast393/1 -> Bus/393  "VINP_CamObsPosXValid10_flg"
  Bus Selector52/4 -> Cast393/1  "<VINP_CamObsPosXValid10_flg_InProc>"
  Bus Selector52/5 -> Cast394/1  "<VINP_CamObsPosY10_m_InProc>"
  Cast394/1 -> Bus/394  "VINP_CamObsPosY10_m"
  Cast395/1 -> Bus/395  "VINP_CamObsPosYValid10_flg"
  Bus Selector52/6 -> Cast395/1  "<VINP_CamObsPosYValid10_flg_InProc>"
  Bus Selector52/7 -> Cast396/1  "<VINP_CamObsVelX10_mps_InProc>"
  Cast396/1 -> Bus/396  "VINP_CamObsVelX10_mps"
  Cast397/1 -> Bus/397  "VINP_CamObsVelXValid10_flg"
  Bus Selector52/8 -> Cast397/1  "<VINP_CamObsVelXValid10_flg_InProc>"
  Cast398/1 -> Bus/398  "VINP_CamObsVelY10_mps"
  Cast399/1 -> Bus/399  "VINP_CamObsVelYValid10_flg"
  Bus Selector52/9 -> Cast398/1  "<VINP_CamObsVelY10_mps_InProc>"
  Bus Selector52/10 -> Cast399/1  "<VINP_CamObsVelYValid10_flg_InProc>"
  Cast400/1 -> Bus/400  "VINP_CamObsAccX10_mps2"
  Cast401/1 -> Bus/401  "VINP_CamObsAccXValid10_flg"
  Bus Selector52/11 -> Cast400/1  "<VINP_CamObsAccX10_mps2_InProc>"
  Bus Selector52/12 -> Cast401/1  "<VINP_CamObsAccXValid10_flg_InProc>"
  Cast402/1 -> Bus/402  "VINP_CamObsAng10_deg"
  Cast403/1 -> Bus/403  "VINP_CamObsAngValid10_flg"
  Bus Selector52/13 -> Cast402/1  "<VINP_CamObsAng10_deg_InProc>"
  Bus Selector52/14 -> Cast403/1  "<VINP_CamObsAngValid10_flg_InProc>"
  Cast404/1 -> Bus/404  "VINP_CamObsAngRate10_degps"
  Cast405/1 -> Bus/405  "VINP_CamObsAngRateValid10_flg"
  Bus Selector52/15 -> Cast404/1  "<VINP_CamObsAngRate10_degps_InProc>"
  Bus Selector52/16 -> Cast405/1  "<VINP_CamObsAngRateValid10_flg_InProc>"
  Cast406/1 -> Bus/406  "VINP_CamObsLength10_m"
  Cast407/1 -> Bus/407  "VINP_CamObsLengthValid10_flg"
  Bus Selector52/17 -> Cast406/1  "<VINP_CamObsLength10_m_InProc>"
  Bus Selector52/18 -> Cast407/1  "<VINP_CamObsLengthValid10_flg_InProc>"
  Cast408/1 -> Bus/408  "VINP_CamObsWidth10_m"
  Cast409/1 -> Bus/409  "VINP_CamObsWidthValid10_flg"
  Bus Selector52/19 -> Cast408/1  "<VINP_CamObsWidth10_m_InProc>"
  Bus Selector52/20 -> Cast409/1  "<VINP_CamObsWidthValid10_flg_InProc>"
  Cast410/1 -> Bus/410  "VINP_CamObsType10_enum"
  Cast411/1 -> Bus/411  "VINP_CamObsTypeValid10_flg"
  Bus Selector52/21 -> Cast410/1  "<VINP_CamObsType10_enum_InProc>"
  Bus Selector52/22 -> Cast411/1  "<VINP_CamObsTypeValid10_flg_InProc>"
  Cast412/1 -> Bus/412  "VINP_CamObsStatus10_enum"
  Cast413/1 -> Bus/413  "VINP_CamObsStatusValid10_flg"
  Bus Selector52/23 -> Cast412/1  "<VINP_CamObsStatus10_enum_InProc>"
  Bus Selector52/24 -> Cast413/1  "<VINP_CamObsStatusValid10_flg_InProc>"
  Cast414/1 -> Bus/414  "VINP_CamObsValid10_enum"
  Cast415/1 -> Bus/415  "VINP_CamObsValidValid10_flg"
  Bus Selector52/25 -> Cast414/1  "<VINP_CamObsValid10_enum_InProc>"
  Bus Selector52/26 -> Cast415/1  "<VINP_CamObsValidValid10_flg_InProc>"
  Cast416/1 -> Bus/416  "VINP_CamObsMoveInO10_enum"
  Cast417/1 -> Bus/417  "VINP_CamObsMoveInOValid10_flg"
  Bus Selector52/27 -> Cast416/1  "<VINP_CamObsMoveInO10_enum_InProc>"
  Bus Selector52/28 -> Cast417/1  "<VINP_CamObsMoveInOValid10_flg_InProc>"
  Cast418/1 -> Bus/418  "VINP_CamObsBlinker10_enum"
  Cast419/1 -> Bus/419  "VINP_CamObsBlinkerValid10_flg"
  Bus Selector52/29 -> Cast418/1  "<VINP_CamObsBlinker10_enum_InProc>"
  Bus Selector52/30 -> Cast419/1  "<VINP_CamObsBlinkerValid10_flg_InProc>"
  Cast420/1 -> Bus/420  "VINP_CamObsBrakeLight10_flg"
  Cast421/1 -> Bus/421  "VINP_CamObsBrakeLightValid10_flg"
  Bus Selector52/31 -> Cast420/1  "<VINP_CamObsBrakeLight10_flg_InProc>"
  Bus Selector52/32 -> Cast421/1  "<VINP_CamObsBrakeLightValid10_flg_InProc>"
  Cast422/1 -> Bus/422  "VINP_CamObsAge10_cnt"
  Cast423/1 -> Bus/423  "VINP_CamObsAgeValid10_flg"
  Bus Selector52/33 -> Cast422/1  "<VINP_CamObsAge10_cnt_InProc>"
  Bus Selector52/34 -> Cast423/1  "<VINP_CamObsAgeValid10_flg_InProc>"
  Cast424/1 -> Bus/424  "VINP_CamObsLane10_enum"
  Cast425/1 -> Bus/425  "VINP_CamObsLaneValid10_flg"
  Bus Selector52/35 -> Cast424/1  "<VINP_CamObsLane10_enum_InProc>"
  Bus Selector52/36 -> Cast425/1  "<VINP_CamObsLaneValid10_flg_InProc>"
  Cast426/1 -> Bus/426  "VINP_CamObsCIPV10_flg"
  Cast427/1 -> Bus/427  "VINP_CamObsCIPVValid10_flg"
  Bus Selector52/37 -> Cast426/1  "<VINP_CamObsCIPV10_flg_InProc>"
  Bus Selector52/38 -> Cast427/1  "<VINP_CamObsCIPVValid10_flg_InProc>"
  Cast428/1 -> Bus/428  "VINP_CamObsReplaced10_flg"
  Cast429/1 -> Bus/429  "VINP_CamObsReplacedValid10_flg"
  Bus Selector52/39 -> Cast428/1  "<VINP_CamObsReplaced10_flg_InProc>"
  Bus Selector52/40 -> Cast429/1  "<VINP_CamObsReplacedValid10_flg_InProc>"
  [EPM_signal_bus] -> Bus Selector53/1
  [EPM_signal_bus] -> Bus Selector54/1
  Cast430/1 -> Bus/318  "VINP_NextLaneC0L_m"
  Bus Selector53/1 -> Cast430/1  "<VINP_NextLaneC0L_m_InHAL>"
  Cast431/1 -> Bus/320  "VINP_NextLaneC1L_rad"
  Cast432/1 -> Bus/319  "VINP_NextLaneC0LValid_flg"
  Bus Selector53/2 -> Cast432/1  "<VINP_NextLaneC0LValid_flg_InHAL>"
  Bus Selector53/3 -> Cast431/1  "<VINP_NextLaneC1L_rad_InHAL>"
  Cast433/1 -> Bus/321  "VINP_NextLaneC1LValid_flg"
  Bus Selector53/4 -> Cast433/1  "<VINP_NextLaneC1LValid_flg_InHAL>"
  Cast434/1 -> Bus/322  "VINP_NextLaneC2L_rat"
  Cast435/1 -> Bus/323  "VINP_NextLaneC2LValid_flg"
  Bus Selector53/5 -> Cast434/1  "<VINP_NextLaneC2L_rat_InHAL>"
  Bus Selector53/6 -> Cast435/1  "<VINP_NextLaneC2LValid_flg_InHAL>"
  Cast436/1 -> Bus/324  "VINP_NextLaneC3L_rat"
  Cast437/1 -> Bus/325  "VINP_NextLaneC3LValid_flg"
  Bus Selector53/7 -> Cast436/1  "<VINP_NextLaneC3L_rat_InHAL>"
  Bus Selector53/8 -> Cast437/1  "<VINP_NextLaneC3LValid_flg_InHAL>"
  Cast438/1 -> Bus/326  "VINP_NextLaneQualityL_enum"
  Cast439/1 -> Bus/327  "VINP_NextLaneQualityLValid_flg"
  Bus Selector53/9 -> Cast438/1  "<VINP_NextLaneQualityL_enum_InHAL>"
  Bus Selector53/10 -> Cast439/1  "<VINP_NextLaneQualityLValid_flg_InHAL>"
  Cast440/1 -> Bus/328  "VINP_NextLaneTypeL_enum"
  Cast441/1 -> Bus/329  "VINP_NextLaneTypeLValid_flg"
  Bus Selector53/11 -> Cast440/1  "<VINP_NextLaneTypeL_enum_InHAL>"
  Bus Selector53/12 -> Cast441/1  "<VINP_NextLaneTypeLValid_flg_InHAL>"
  Cast442/1 -> Bus/330  "VINP_NextLaneMrkWidL_m"
  Cast443/1 -> Bus/331  "VINP_NextLaneMrkWidLValid_flg"
  Bus Selector53/13 -> Cast442/1  "<VINP_NextLaneMrkWidL_m_InHAL>"
  Bus Selector53/14 -> Cast443/1  "<VINP_NextLaneMrkWidLValid_flg_InHAL>"
  Cast444/1 -> Bus/332  "VINP_NextViewRangeStartL_m"
  Cast445/1 -> Bus/333  "VINP_NextViewRangeStartLValid_flg"
  Bus Selector53/15 -> Cast444/1  "<VINP_NextViewRangeStartL_m_InHAL>"
  Bus Selector53/16 -> Cast445/1  "<VINP_NextViewRangeStartLValid_flg_InHAL>"
  Cast446/1 -> Bus/334  "VINP_NextViewRangeEndL_m"
  Cast447/1 -> Bus/335  "VINP_NextViewRangeEndLValid_flg"
  Bus Selector53/17 -> Cast446/1  "<VINP_NextViewRangeEndL_m_InHAL>"
  Bus Selector53/18 -> Cast447/1  "<VINP_NextViewRangeEndLValid_flg_InHAL>"
  Cast448/1 -> Bus/336  "VINP_NextLaneC0R_m"
  Cast449/1 -> Bus/338  "VINP_NextLaneC1R_rad"
  Cast450/1 -> Bus/337  "VINP_NextLaneC0RValid_flg"
  Cast451/1 -> Bus/339  "VINP_NextLaneC1RValid_flg"
  Cast452/1 -> Bus/340  "VINP_NextLaneC2R_rat"
  Cast453/1 -> Bus/341  "VINP_NextLaneC2RValid_flg"
  Cast454/1 -> Bus/342  "VINP_NextLaneC3R_rat"
  Cast455/1 -> Bus/343  "VINP_NextLaneC3RValid_flg"
  Cast456/1 -> Bus/344  "VINP_NextLaneQualityR_enum"
  Cast457/1 -> Bus/345  "VINP_NextLaneQualityRValid_flg"
  Cast458/1 -> Bus/346  "VINP_NextLaneTypeR_enum"
  Cast459/1 -> Bus/347  "VINP_NextLaneTypeRValid_flg"
  Cast460/1 -> Bus/348  "VINP_NextLaneMrkWidR_m"
  Cast461/1 -> Bus/349  "VINP_NextLaneMrkWidRValid_flg"
  Cast462/1 -> Bus/350  "VINP_NextViewRangeStartR_m"
  Cast463/1 -> Bus/351  "VINP_NextViewRangeStartRValid_flg"
  Cast464/1 -> Bus/352  "VINP_NextViewRangeEndR_m"
  Cast465/1 -> Bus/353  "VINP_NextViewRangeEndRValid_flg"
  Bus Selector54/1 -> Cast448/1  "<VINP_NextLaneC0R_m_InHAL>"
  Bus Selector54/4 -> Cast451/1  "<VINP_NextLaneC1RValid_flg_InHAL>"
  Bus Selector54/17 -> Cast464/1  "<VINP_NextViewRangeEndR_m_InHAL>"
  Bus Selector54/13 -> Cast460/1  "<VINP_NextLaneMrkWidR_m_InHAL>"
  Bus Selector54/6 -> Cast453/1  "<VINP_NextLaneC2RValid_flg_InHAL>"
  Bus Selector54/2 -> Cast450/1  "<VINP_NextLaneC0RValid_flg_InHAL>"
  Bus Selector54/14 -> Cast461/1  "<VINP_NextLaneMrkWidRValid_flg_InHAL>"
  Bus Selector54/5 -> Cast452/1  "<VINP_NextLaneC2R_rat_InHAL>"
  Bus Selector54/3 -> Cast449/1  "<VINP_NextLaneC1R_rad_InHAL>"
  Bus Selector54/11 -> Cast458/1  "<VINP_NextLaneTypeR_enum_InHAL>"
  Bus Selector54/9 -> Cast456/1  "<VINP_NextLaneQualityR_enum_InHAL>"
  Bus Selector54/7 -> Cast454/1  "<VINP_NextLaneC3R_rat_InHAL>"
  Bus Selector54/16 -> Cast463/1  "<VINP_NextViewRangeStartRValid_flg_InHAL>"
  Bus Selector54/12 -> Cast459/1  "<VINP_NextLaneTypeRValid_flg_InHAL>"
  Bus Selector54/15 -> Cast462/1  "<VINP_NextViewRangeStartR_m_InHAL>"
  Bus Selector54/18 -> Cast465/1  "<VINP_NextViewRangeEndRValid_flg_InHAL>"
  Bus Selector54/10 -> Cast457/1  "<VINP_NextLaneQualityRValid_flg_InHAL>"
  Bus Selector54/8 -> Cast455/1  "<VINP_NextLaneC3RValid_flg_InHAL>"
